simulink model "MCVT_Integration"  (R2020a) (continued)
== system MCVT_Integration/Sys5 - ECLSS/Active Thermal Control System (ATCS) == (continued)
  PID Motor speed Control : simulink/Continuous/PID Controller  Controller=PID  Form=Parallel  TimeDomain=Discrete-time  UseExternalTs=off  SampleTime=dt_5  IntegratorMethod=Backward Euler  FilterMethod=Forward Euler  ControllerParametersSource=internal  P=200  I=20  D=0  UseFilter=off  N=100  TunerSelectOption=Transfer Function Based (PID Tuner App)  InitialConditionSource=internal  InitialConditionForIntegrator=1  InitialConditionForFilter=0  DifferentiatorICPrevScaledInput=0  ExternalReset=none  TrackingMode=off  Kt=1  LimitOutput=on  UpperSaturationLimit=120  LowerSaturationLimit=0  LinearizeAsGain=off  AntiWindupMode=none  Kb=1  POutMin=[]  POutMax=[]  IOutMin=[]  IOutMax=[]  DOutMin=[]  DOutMax=[]  NOutMin=[]  NOutMax=[]  SumOutMin=[]  SumOutMax=[]  SaturationOutMin=[]  SaturationOutMax=[]  PParamMin=[]  PParamMax=[]  IParamMin=[]  IParamMax=[]  DParamMin=[]  DParamMax=[]  NParamMin=[]  NParamMax=[]  KbParamMin=[]  KbParamMax=[]  KtParamMin=[]  KtParamMax=[]  KbOutMin=[]  KbOutMax=[]  KtOutMin=[]  KtOutMax=[]  IntegratorOutMin=[]  IntegratorOutMax=[]  FilterOutMin=[]  FilterOutMax=[]  SumI1OutMin=[]  SumI1OutMax=[]  SumI2OutMin=[]  SumI2OutMax=[]  SumI3OutMin=[]  SumI3OutMax=[]  SumI4OutMin=[]  SumI4OutMax=[]  SumDOutMin=[]  SumDOutMax=[]  DifferentiatorOutMin=[]  DifferentiatorOutMax=[]  FilterDiffOutCoefMin=[]  FilterDiffOutCoefMax=[]  ReciprocalOutMin=[]  ReciprocalOutMax=[]  SumDenOutMin=[]  SumDenOutMax=[]  SumNumOutMin=[]  SumNumOutMax=[]  DivideOutMin=[]  DivideOutMax=[]  UdiffTsProdOutMin=[]  UdiffTsProdOutMax=[]  NTsProdOutMin=[]  NTsProdOutMax=[]  UintegralTsProdOutMin=[]  UintegralTsProdOutMax=[]  UngainTsProdOutMin=[]  UngainTsProdOutMax=[]  IntegratorContinuousStateAttributes=''  IntegratorStateMustResolveToSignalObject=off  IntegratorRTWStateStorageClass=Auto  FilterContinuousStateAttributes=''  FilterStateMustResolveToSignalObject=off  FilterRTWStateStorageClass=Auto  ParallelPVariant=InternalParameters  IdealPVariant=Disabled  IVariant=InternalParameters  DVariant=InternalParameters  IntegratorVariant=Discrete  SatVariant=Enabled  AWVariant=Passthrough  PCopyVariant=Disabled  TRVariant=Disabled  FdbkBlocksVariant=Forward  IdealPFdbkVariant=Disabled  SatFdbkVariant=Disabled  DerivativeFilterVariant=Differentiator  NVariant=Passthrough  NCopyVariant=Disabled_SignalSpec  FilterICVariant=InternalICsDifferentiator  IntegratorICVariant=InternalICs  ExternalResetVariant=Disabled  TRSumVariant=Passthrough  SumFdbkVariant=Disabled  SumVariant=Sum_PID  TsampFilterVariant=InternalTs  TsampNgainVariant=Passthrough  TsampIntegralVariant=Passthrough
  Scope : Scope  Commented=on  NumInputPorts=2
  Scope1 : Scope  Commented=on
  Scope2 : Scope  Commented=on
  Scope3 : Scope  Commented=on
  Scope4 : Scope
  Sensors : MATLAB Function  TreatAsAtomicUnit=on
  Sensors1 : MATLAB Function  TreatAsAtomicUnit=on
  Sum : Sum  Inputs=+-
  Sum1 : Sum  Inputs=|+-
  Sum2 : Sum
  Sys5_int_air_properties : Constant  Value=input_air
  Sys5_int_compressor_parameters : Constant  Value=input_CP
  Sys5_int_condenser_parameters : Constant  Value=input_Cond
  Sys5_int_discretization_information : Constant  Value=input_discretization
  Sys5_int_evaporator_parameters : Constant  Value=input_Evap
  Sys5_int_expansion_vavle_parameters : Constant  Value=input_EV
  Sys5_int_radiator_loop_parameters : Constant  Value=input_radiatorLoop
  Variable set temperature : MATLAB Function  TreatAsAtomicUnit=on  SystemSampleTime=dt_5
  Vavle opening control : MATLAB Function  TreatAsAtomicUnit=on
  on and off dynamic enforcer : MATLAB Function  TreatAsAtomicUnit=on
  tempadjust : MATLAB Function  TreatAsAtomicUnit=on  SystemSampleTime=dt_5
  Sys5_out_sensor_data_Tfs [C] : Outport  VectorParamsAs1DForOutWhenUnconnected=off
  Sys5_out_sensor_data_Trs [C] : Outport  Port=2  VectorParamsAs1DForOutWhenUnconnected=off
  Sys5_out_power_request [kW] : Outport  Port=3
  Sys5_out_power consumption [kW] : Outport  Port=4  VectorParamsAs1DForOutWhenUnconnected=off
  Sys5_out_heating_energy [kW] : Outport  Port=5  VectorParamsAs1DForOutWhenUnconnected=off
  Sys5_out_airflow [m^3/s] : Outport  Port=6  VectorParamsAs1DForOutWhenUnconnected=off
  Sys5_out_evaporator temperature [C] : Outport  Port=7  VectorParamsAs1DForOutWhenUnconnected=off
  "Sys5_out_paint Health State (0: clean, 1: full damage)" : Outport  Port=8
  "Sys5_out_dust Health State (0: clean, 1: full damage)" : Outport  Port=9
  Sys5_Out_Damage_Information : Outport  Port=10
  wires:
  Sys5_int_evaporator_parameters/1 -> ATCS/4(input_Evap)
  Sys5_int_compressor_parameters/1 -> ATCS/6(input_CP)
  Sys5_int_condenser_parameters/1 -> ATCS/5(input_Cond)
  ATCS/12(Ye) -> Memory9/1
  Memory1/1 -> ATCS/1(Xk)
  Sys5_int_radiator_loop_parameters/1 -> ATCS/8(input_RL)
  Sys5_int_discretization_information/1 -> ATCS/3(input_discretization)
  Sys5_int_air_properties/1 -> ATCS/7(input_air)
  ATCS/1(X) -> Memory1/1
  Sum/1 -> PID Motor speed Control/1
  Sys5_in_solar radiation [W/m^2]/1 -> [A]
  ATCS/9(Tair_out) -> Sys5_out_evaporator temperature [C]/1
  Vavle opening control/1(input_EV) -> ATCS/14(input_EV)
  Liquid fraction setpoint/1 -> Vavle opening control/2(Ye_set)
  Memory9/1 -> Vavle opening control/1(Ye)
  Sys5_int_expansion_vavle_parameters/1 -> Vavle opening control/3("input_EV ")
  Variable set temperature/2(set) -> Memory4/1
  Memory4/1 -> Variable set temperature/5("set ")
  Faulty Panels/3(faulty panels) -> ATCS/9(input_dust)
  ATCS/3(Pc) -> on and off dynamic enforcer/2(Pc)
  ATCS/4(Tfs) -> Sensors/1(Tfs)
  Sensors/1(TTfs) -> Sys5_out_sensor_data_Tfs [C]/1
  ATCS/5(Trs) -> Sensors1/1(Trs)
  Sensors1/1(TTrs) -> Sys5_out_sensor_data_Trs [C]/1
  Heater_capacity (kW)/1 -> MATLAB Function6/3(Qh)
  ATCS/10(WCP) -> Add/1, Scope2/1, Sum2/1, Variable set temperature/1(Pd)
  MATLAB Function6/2(Ph) -> [D]
  Add/1 -> Sys5_out_power consumption [kW]/1
  PID Motor speed Control/1 -> ATCS/13(nCP), Scope1/1
  Sys5_in_airflow through the evaporator [m^3/s]/1 -> ATCS/12(Vair_in)
  Variable set temperature/1(Tset) -> Memory2/1, Memory3/1
  on and off dynamic enforcer/1(Vop) -> Memory6/1
  ATCS/2(Pe) -> on and off dynamic enforcer/1(Pe)
  Memory6/1 -> ATCS/2(Vop)
  Faulty Panels/1("Paint Health State(0: clean, 1:full damage)") -> [F]
  Faulty Panels/2("Dust Health State (0: clean, 1:full damage)") -> [G]
  "Sys5_in_dust repairing agent (1:active, 0:inactive)"/1 -> Faulty Panels/2("Dust repairing agent (1: active, 0: inactive)")
  "Sys5_in_paint repairing agent (1:active, 0:inactive)"/1 -> Faulty Panels/3("Paint repairing agent (1: active, 0: inactive)")
  Sum1/1 -> Heater PID Controller/1
  Heater PID Controller/1 -> MATLAB Function6/1(Ps)
  Sys5_in_limited power supply [kW]/1 -> MATLAB Function6/2(lim_supply), Variable set temperature/3(Ps), Scope4/1
  Memory3/1 -> Variable set temperature/4("Tset ")
  Sys5_in_impact (1-4)/1 -> Faulty Panels/4(Impact)
  MATLAB Function6/1(P_request) -> Sum2/2
  Sum2/1 -> Sys5_out_power_request [kW]/1
  Sys5_in_Tset [C]/1 -> tempadjust/2(Tset), Variable set temperature/2(Tset0)
  Fan switch/1 -> [E]
  Gain/1 -> Faulty Panels/1(Sys5_in_Dust deposition rate (g/cm^2))
  Sys5_in_indoor temperature [C]/1 -> tempadjust/1(x)  "Indoor Temp"
  Clock/1 -> tempadjust/3(t)
  Discrete-Time Integrator/1 -> Gain/1
  Sys5_in_dust_Input [g/cm^2/s]/1 -> Discrete-Time Integrator/1
  Fan speed on (m^3/s)/1 -> Fan switch/1
  Fan speed off (m^3/s)/1 -> Fan switch/3
  [A] -> ATCS/10(Fs)
  tempadjust/1(y) -> [B]  "Indoor Temp"
  [B] -> ATCS/11(Tair_in)
  [B] -> Scope/2, Sum/1
  [B] -> Sum1/2
  [B] -> Fan switch/2
  Memory2/1 -> [C]  "Tset"
  [C] -> Sum1/1
  [C] -> Scope/1, Sum/2
  [D] -> Sys5_out_heating_energy [kW]/1
  [D] -> Scope3/1, Add/2
  [E] -> Sys5_out_airflow [m^3/s]/1
  [F] -> "Sys5_out_paint Health State (0: clean, 1: full damage)"/1
  [G] -> "Sys5_out_dust Health State (0: clean, 1: full damage)"/1
  Sys5_Repair_Status/1 -> Bus Selector/1
  Bus Selector/1 -> [Repair_ECLSS_Dust]  "<Repair_ECLSS_Dust>"
  Bus Selector/2 -> [Repair_ECLSS_Paint]  "<Repair_ECLSS_Paint>"
  [Repair_ECLSS_Dust] -> Faulty Panels/5(Repair_ECLSS_Dust)
  [Repair_ECLSS_Paint] -> Faulty Panels/6(Repair_ECLSS_Paint)
  Faulty Panels/4(Sys5_Out_Damage_Information) -> [Sys5_Out_Damage_Information]
  [Sys5_Out_Damage_Information] -> Sys5_Out_Damage_Information/1

== system MCVT_Integration/Sys5 - ECLSS/Human//System 5 ==
  dt : Inport
  activity_id : Inport  Port=2
  Location_id : Inport  Port=3
  Human Heat Model V2 : MATLAB Function  TreatAsAtomicUnit=on
  heat_profile_final : Outport
  r : Outport  Port=2
  theta : Outport  Port=3
  wires:
  Human Heat Model V2/1(heat_profile_final) -> heat_profile_final/1
  Location_id/1 -> Human Heat Model V2/3(Location_id)
  Human Heat Model V2/2(r) -> r/1
  Human Heat Model V2/3(theta) -> theta/1
  dt/1 -> Human Heat Model V2/1(dt)
  activity_id/1 -> Human Heat Model V2/2(activity_id)

== system MCVT_Integration/Sys5 - ECLSS/Interior Pressure Control System (IPCS) ==
  note: Inputs
  note: Controller
  note: Outputs
  Sys5_in_setpoint_pressure [Pa] : Inport
  Sys 5_in_interior pressure [Pa] : Inport  Port=2
  Sys5_in_faulty_valve [-] : Inport  Port=3
  Air storage tank volume : Constant  Value=ECLSS_Vst
  Area of Flow Restrictor : Constant  Value=ECLSS_IPS_restrictor.cross_area
  Area of Relief valve : Constant  Value=Aref
  Gain1 : Gain  Gain=-1
  High pressure stored air : MATLAB Function  TreatAsAtomicUnit=on  SystemSampleTime=dt_5
  Memory5 : Memory  InitialCondition=Ps0  InheritSampleTime=on
  Memory6 : Memory  InheritSampleTime=on
  "PID Controller Storage Control Valve " : simulink/Continuous/PID Controller  Controller=PID  Form=Parallel  TimeDomain=Discrete-time  UseExternalTs=off  SampleTime=dt_5  IntegratorMethod=Forward Euler  FilterMethod=Forward Euler  ControllerParametersSource=internal  P=1e-10  I=1e-11  D=0  UseFilter=on  N=100  TunerSelectOption=Transfer Function Based (PID Tuner App)  InitialConditionSource=internal  InitialConditionForIntegrator=0  InitialConditionForFilter=0  DifferentiatorICPrevScaledInput=0  ExternalReset=none  TrackingMode=off  Kt=1  LimitOutput=off  UpperSaturationLimit=A_flow_restrictor  LowerSaturationLimit=0  LinearizeAsGain=on  AntiWindupMode=none  Kb=1  POutMin=[]  POutMax=[]  IOutMin=[]  IOutMax=[]  DOutMin=[]  DOutMax=[]  NOutMin=[]  NOutMax=[]  SumOutMin=[]  SumOutMax=[]  SaturationOutMin=[]  SaturationOutMax=[]  PParamMin=[]  PParamMax=[]  IParamMin=[]  IParamMax=[]  DParamMin=[]  DParamMax=[]  NParamMin=[]  NParamMax=[]  KbParamMin=[]  KbParamMax=[]  KtParamMin=[]  KtParamMax=[]  KbOutMin=[]  KbOutMax=[]  KtOutMin=[]  KtOutMax=[]  IntegratorOutMin=[]  IntegratorOutMax=[]  FilterOutMin=[]  FilterOutMax=[]  SumI1OutMin=[]  SumI1OutMax=[]  SumI2OutMin=[]  SumI2OutMax=[]  SumI3OutMin=[]  SumI3OutMax=[]  SumI4OutMin=[]  SumI4OutMax=[]  SumDOutMin=[]  SumDOutMax=[]  DifferentiatorOutMin=[]  DifferentiatorOutMax=[]  FilterDiffOutCoefMin=[]  FilterDiffOutCoefMax=[]  ReciprocalOutMin=[]  ReciprocalOutMax=[]  SumDenOutMin=[]  SumDenOutMax=[]  SumNumOutMin=[]  SumNumOutMax=[]  DivideOutMin=[]  DivideOutMax=[]  UdiffTsProdOutMin=[]  UdiffTsProdOutMax=[]  NTsProdOutMin=[]  NTsProdOutMax=[]  UintegralTsProdOutMin=[]  UintegralTsProdOutMax=[]  UngainTsProdOutMin=[]  UngainTsProdOutMax=[]  IntegratorContinuousStateAttributes=''  IntegratorStateMustResolveToSignalObject=off  IntegratorRTWStateStorageClass=Auto  FilterContinuousStateAttributes=''  FilterStateMustResolveToSignalObject=off  FilterRTWStateStorageClass=Auto  ParallelPVariant=InternalParameters  IdealPVariant=Disabled  IVariant=InternalParameters  DVariant=InternalParameters  IntegratorVariant=Discrete  SatVariant=Disabled  AWVariant=Passthrough  PCopyVariant=Disabled  TRVariant=Disabled  FdbkBlocksVariant=Forward  IdealPFdbkVariant=Disabled  SatFdbkVariant=Disabled  DerivativeFilterVariant=ForwardEulerFilter  NVariant=InternalParameters  NCopyVariant=Disabled  FilterICVariant=InternalICsFilter  IntegratorICVariant=InternalICs  ExternalResetVariant=Disabled  TRSumVariant=Passthrough  SumFdbkVariant=Disabled  SumVariant=Sum_PID  TsampFilterVariant=InternalTs  TsampNgainVariant=Passthrough  TsampIntegralVariant=Passthrough
  Power consumption Calculator : MATLAB Function  TreatAsAtomicUnit=on
  Relief Valve Control : MATLAB Function  TreatAsAtomicUnit=on
  Restrictor : MATLAB Function  TreatAsAtomicUnit=on
  Saturation : Saturate  UpperLimit=ECLSS_IPS_restrictor.cross_area  LowerLimit=0
  Saturation1 : Saturate  UpperLimit=Aref  LowerLimit=0
  Scope : Scope
  Scope1 : Scope  NumInputPorts=2
  Scope2 : Scope  NumInputPorts=2
  Scope3 : Scope  NumInputPorts=2
  Sum : Sum  Inputs=|+-
  Sys5.2_int_valve parameter : Constant  Value=Kref
  Timestep for system 5 : Constant  Value=dt_5
  Sys5_out_air_released [kg/s] : Outport  VectorParamsAs1DForOutWhenUnconnected=off
  Sys5_out_air_supply [kg/s] : Outport  Port=2  VectorParamsAs1DForOutWhenUnconnected=off
  Sys5_out_consumed_power [kW] : Outport  Port=3
  wires:
  Power consumption Calculator/1(power) -> Sys5_out_consumed_power [kW]/1
  Saturation/1 -> Power consumption Calculator/1(Fr_now), Scope2/1, Restrictor/2(Afr)  "effective Cross-sectional Area"
  Area of Relief valve/1 -> Scope3/2, Power consumption Calculator/4(Rel_100)
  "PID Controller Storage Control Valve "/1 -> Saturation/1, Scope/1, Gain1/1
  Sys5.2_int_valve parameter/1 -> Relief Valve Control/2(Kref)
  Sum/1 -> "PID Controller Storage Control Valve "/1
  Saturation1/1 -> Scope3/1, Power consumption Calculator/3(Rel_now), Relief Valve Control/1(Aref)  "effective Cross-sectional Area"
  High pressure stored air/1(Ps) -> Memory5/1, Restrictor/1(Ps)
  Memory6/1 -> High pressure stored air/3(ms)
  Area of Flow Restrictor/1 -> Scope2/2, Power consumption Calculator/2(Fr_100)
  Memory5/1 -> High pressure stored air/1(Psk)
  Timestep for system 5/1 -> High pressure stored air/2(dt)
  Relief Valve Control/1(m_rel) -> Sys5_out_air_released [kg/s]/1
  Gain1/1 -> Saturation1/1
  Sys5_in_faulty_valve [-]/1 -> [B]
  Sys5_in_setpoint_pressure [Pa]/1 -> Sum/1, Scope1/1
  Air storage tank volume/1 -> High pressure stored air/4(ECLSS_Vst)
  Restrictor/1(ms) -> Sys5_out_air_supply [kg/s]/1, Memory6/1
  Sys 5_in_interior pressure [Pa]/1 -> [A]
  [A] -> Scope1/2, Sum/2
  [A] -> Relief Valve Control/3(Pint)
  [A] -> Restrictor/3(Pint)
  [B] -> Restrictor/4(Vop)

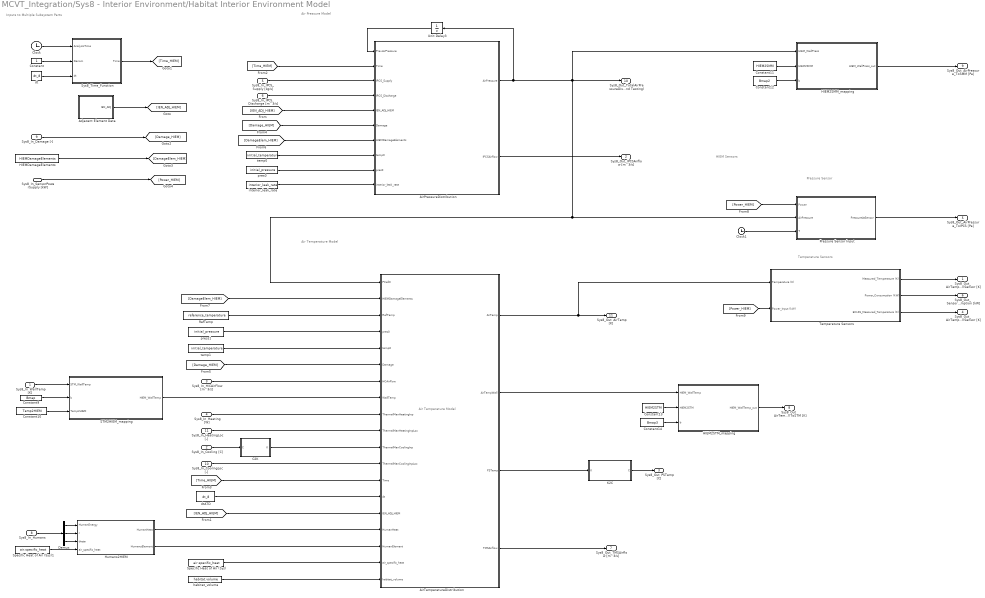
== system MCVT_Integration/Sys8 - Interior Environment/Habitat Interior Environment Model ==
  note: HIEM Sensors
  note: Inputs to Multiple Subsystem Parts
  note: Air Temperature Model
  note: Air Pressure Model
  note: Air Temperature Model
  note: Temperature Sensors
  note: Pressure Sensor
  Sys8_In_WallTemp [K] : Inport
  Sys8_In_Cooling [C] : Inport  Port=2
  Sys8_In_H/CAirFlow [m^3/s] : Inport  Port=3
  Sys8_In_Heating [W] : Inport  Port=4
  Sys8_In_IPCS_Supply [kg/s] : Inport  Port=5
  Sys8_In_IPCS_Discharge [m^3/s] : Inport  Port=6
  Sys8_In_SensorPowerSupply [kW] : Inport  Port=7
  Sys8_In_Humans : Inport  Port=8
  Sys8_In_Damage [-] : Inport  Port=9
  Sys8_In_CoolingLoc [-] : Inport  Port=10
  Sys8_In_HeatingLoc [-] : Inport  Port=11
  Adjacent Element Data : MATLAB Function  TreatAsAtomicUnit=on  SystemSampleTime=dt_8
  AirPressureDistribution : MATLAB Function  TreatAsAtomicUnit=on
  AirTemperatureDistribution : MATLAB Function  TreatAsAtomicUnit=on
  C2K : MATLAB Function  TreatAsAtomicUnit=on
  Clock : Clock
      settings: Decimation=5
  Clock1 : Clock
  Constant : Constant  SampleTime=dt_8
  Constant10 : Constant  Value=Temp2HIEM
  Constant11 : Constant  Value=HIEM2SMM
  Constant12 : Constant  Value=Bmap2
  Constant13 : Constant  Value=HIEM2STM
  Constant14 : Constant  Value=Bmap3
  Constant9 : Constant  Value=Bmap
  Demux : Demux  Outputs=3
  HIEM2SMM_mapping : MATLAB Function  TreatAsAtomicUnit=on
  HIEM2STM_mapping : MATLAB Function  TreatAsAtomicUnit=on
  HIEMDamageElements : Constant  Value=HIEMDamageElements
  Humans2HIEM : MATLAB Function  TreatAsAtomicUnit=on
  K2C : MATLAB Function  TreatAsAtomicUnit=on
  Pressure Sensor Input : MATLAB Function  TreatAsAtomicUnit=on  SystemSampleTime=dt_8
  RefTemp : Constant  Value=reference_temperature
  STM2HIEM_mapping : MATLAB Function  TreatAsAtomicUnit=on
  Specific Heat of Air (Sys) : Constant  Value=air.specific_heat
  Specific Heat of Air (Sys)1 : Constant  Value=air.specific_heat
  Sys8_Time_Function : MATLAB Function  TreatAsAtomicUnit=on  SystemSampleTime=dt_8
  Temperature Sensors : Subsystem
  Unit Delay3 : UnitDelay  InitialCondition=ones(112,1)*100000  SampleTime=dt_8  HasFrameUpgradeWarning=on
  dt : Constant  Value=dt_8
  dtATD : Constant  Value=dt_8
  habitat_volume : Constant  Value=habitat.volume
  interior_leak_rate : Constant  Value=interior_leak_rate
  pres0 : Constant  Value=initial_pressure
  press1 : Constant  Value=initial_pressure
  temp0 : Constant  Value=initial_temperature
  temp1 : Constant  Value=initial_temperature
  Sys8_Out_AirTempWallSensor [K] : Outport  PortDimensions=[3 1]  VectorParamsAs1DForOutWhenUnconnected=off
  Sys8_Out_IPCSAirflow [m^3/s] : Outport  Port=2  VectorParamsAs1DForOutWhenUnconnected=off
  Sys8_Out_PSTemp [C] : Outport  Port=3  OutDataTypeStr=double  PortDimensions=1  VectorParamsAs1DForOutWhenUnconnected=off
  Sys8_Out_AirTempECLSSWallSensor [K] : Outport  Port=4  VectorParamsAs1DForOutWhenUnconnected=off
  Sys8_Out_AirPressure_ToIPCS [Pa] : Outport  Port=5  VectorParamsAs1DForOutWhenUnconnected=off
  Sys8_Out_SensorPowerConsumption [kW] : Outport  Port=6  VectorParamsAs1DForOutWhenUnconnected=off
  Sys8_Out_TMSAirflow [m^3/s] : Outport  Port=7  VectorParamsAs1DForOutWhenUnconnected=off
  Sys8_Out_AirTempWallToSTM [K] : Outport  Port=8  VectorParamsAs1DForOutWhenUnconnected=off
  Sys8_Out_AirPressure_ToSMM [Pa] : Outport  Port=9  VectorParamsAs1DForOutWhenUnconnected=off
  Sys8_Out_TotalAirPressureDistribution (Plotting and Testing) : Outport  Port=10
  Sys8_Out_AirTemp [K] : Outport  Port=11  VectorParamsAs1DForOutWhenUnconnected=off
  wires:
  Clock/1 -> Sys8_Time_Function/1(AnalysisTime)
  Constant/1 -> Sys8_Time_Function/2(Denom)
  dt/1 -> Sys8_Time_Function/3(dt)
  Sys8_In_Cooling [C]/1 -> C2K/1(C)
  Sys8_In_WallTemp [K]/1 -> STM2HIEM_mapping/1(STM_WallTemp)
  Unit Delay3/1 -> AirPressureDistribution/1(PrevAirPressure)
  Sys8_In_CoolingLoc [-]/1 -> AirTemperatureDistribution/12(ThermalManCoolingInpLoc)
  Sys8_In_IPCS_Supply [kg/s]/1 -> AirPressureDistribution/3(IPCS_Supply)
  Sys8_In_IPCS_Discharge [m^3/s]/1 -> AirPressureDistribution/4(IPCS_Discharge)
  Pressure Sensor Input/1(PressureAtSensor) -> Sys8_Out_AirPressure_ToIPCS [Pa]/1
  C2K/1(K) -> AirTemperatureDistribution/11(ThermalManCoolingInp)
  K2C/1(C) -> Sys8_Out_PSTemp [C]/1
  dtATD/1 -> AirTemperatureDistribution/14(dt)
  AirTemperatureDistribution/1(AirTemp) -> Sys8_Out_AirTemp [K]/1, Temperature Sensors/1(Temperature (K))
  AirTemperatureDistribution/3(PSTemp) -> K2C/1(K)
  AirTemperatureDistribution/2(AirTempWall) -> HIEM2STM_mapping/1(HIEM_WallTemp)
  AirPressureDistribution/2(IPCSAirflow) -> Sys8_Out_IPCSAirflow [m^3/s]/1
  AirPressureDistribution/1(AirPressure) -> Unit Delay3/1, HIEM2SMM_mapping/1(HIEM_WallPress), Sys8_Out_TotalAirPressureDistribution (Plotting and Testing)/1, AirTemperatureDistribution/1(PCell0), Pressure Sensor Input/2(AirPressure)
  Sys8_In_H/CAirFlow [m^3/s]/1 -> AirTemperatureDistribution/7(HCAirFlow)
  Adjacent Element Data/1(IEN_ADJ) -> [IEN_ADJ_HIEM]
  Sys8_In_SensorPowerSupply [kW]/1 -> [Power_HIEM]
  Temperature Sensors/1(Measured_Temperature (K)) -> Sys8_Out_AirTempWallSensor [K]/1
  Temperature Sensors/2(Power_Consumption (kW)) -> Sys8_Out_SensorPowerConsumption [kW]/1
  AirTemperatureDistribution/4(TMSAirflow) -> Sys8_Out_TMSAirflow [m^3/s]/1
  HIEM2SMM_mapping/1(HIEM_WallPress_out) -> Sys8_Out_AirPressure_ToSMM [Pa]/1
  STM2HIEM_mapping/1(HIEM_WallTemp) -> AirTemperatureDistribution/8(WallTemp)
  HIEM2STM_mapping/1(HIEM_WallTemp_out) -> Sys8_Out_AirTempWallToSTM [K]/1
  Sys8_In_Humans/1 -> Demux/1
  Demux/2 -> Humans2HIEM/2(r)
  Demux/3 -> Humans2HIEM/3(theta)
  Demux/1 -> Humans2HIEM/1(HumanEnergy)
  Humans2HIEM/1(HumanHeat) -> AirTemperatureDistribution/16(HumanHeat)
  Humans2HIEM/2(HumansElement) -> AirTemperatureDistribution/17(HumanElement)
  Sys8_In_Heating [W]/1 -> AirTemperatureDistribution/9(ThermalManHeatingInp)
  Sys8_In_HeatingLoc [-]/1 -> AirTemperatureDistribution/10(ThermalManHeatingInpLoc)
  Sys8_Time_Function/1(Time) -> [Time_HIEM]
  Sys8_In_Damage [-]/1 -> [Damage_HIEM]
  Temperature Sensors/3(ECLSS_Measured_Temperature (K)) -> Sys8_Out_AirTempECLSSWallSensor [K]/1
  temp0/1 -> AirPressureDistribution/8(temp0)
  pres0/1 -> AirPressureDistribution/9(pres0)
  temp1/1 -> AirTemperatureDistribution/5(temp0)
  RefTemp/1 -> AirTemperatureDistribution/3(RefTemp)
  Constant9/1 -> STM2HIEM_mapping/2(b)
  Constant10/1 -> STM2HIEM_mapping/3(Temp2HIEM)
  Constant11/1 -> HIEM2SMM_mapping/2(HIEM2SMM)
  Constant12/1 -> HIEM2SMM_mapping/3(b)
  Constant14/1 -> HIEM2STM_mapping/3(b)
  Constant13/1 -> HIEM2STM_mapping/2(HIEM2STM)
  Clock1/1 -> Pressure Sensor Input/3(T)
  interior_leak_rate/1 -> AirPressureDistribution/10(interior_leak_rate)
  HIEMDamageElements/1 -> [DamageElem_HIEM]
  habitat_volume/1 -> AirTemperatureDistribution/19(habitat_volume)
  press1/1 -> AirTemperatureDistribution/4(pres0)
  Specific Heat of Air (Sys)/1 -> AirTemperatureDistribution/18(air_specific_heat)
  Specific Heat of Air (Sys)1/1 -> Humans2HIEM/4(air_specific_heat)
  [IEN_ADJ_HIEM] -> AirPressureDistribution/5(IEN_ADJ_HIEM)
  [IEN_ADJ_HIEM] -> AirTemperatureDistribution/15(IEN_ADJ_HIEM)
  [Time_HIEM] -> AirPressureDistribution/2(Time)
  [Time_HIEM] -> AirTemperatureDistribution/13(Time)
  [Damage_HIEM] -> AirPressureDistribution/6(Damage)
  [Damage_HIEM] -> AirTemperatureDistribution/6(Damage)
  [DamageElem_HIEM] -> AirPressureDistribution/7(HIEMDamageElements)
  [DamageElem_HIEM] -> AirTemperatureDistribution/2(HIEMDamageElements)
  [Power_HIEM] -> Temperature Sensors/2(Power_Input (kW))
  [Power_HIEM] -> Pressure Sensor Input/1(Power)

== system MCVT_Integration/Sys9 - Exterior Environment/Exterior Environment ==
  note: Moonquake
  note: Solar Irradiation
  note: Dust Accumulation
  note: Meteoroid Impact
  Sys9_in_Time : Inport
  Sys9_in_InclinationAngle : Inport  Port=2
  Sys9_in_Magnitude : Inport  Port=3
  3D Solar Irradiation model (v9) 1 : MATLAB Function  TreatAsAtomicUnit=on
  Constant6 : Constant  Value=0
  Delay : Delay  DelayLength=Sys9_int_EqStart/dt_9  InitialCondition=0
  Delay1 : Delay  DelayLength=Sys9_int_EqStart/dt_9  InitialCondition=0
  Demux : Demux  Outputs=3
  Determine_Damage_Case : MATLAB Function  TreatAsAtomicUnit=on
  Dust : Subsystem
  ExhaustEvent : Subsystem
  Impact_Location : MATLAB Function  TreatAsAtomicUnit=on
  Landing Pad Parameters1 : Constant  Value=Sys9_int_L  SampleTime=dt_9
  MATLAB Function : MATLAB Function  TreatAsAtomicUnit=on
  Mapping function from Solar Irradiation to SPLT and STM : MATLAB Function  TreatAsAtomicUnit=on
  MeteoroidEvent : Subsystem
  Reads cartesian coordinates : MATLAB Function  TreatAsAtomicUnit=on
  SR_STM_ei_th : ToWorkspace  VariableName=SR_STM_ei_th  SampleTime=-1
  SR_STM_ei_th2 : ToWorkspace  VariableName=SR_STM_ei_th2  SampleTime=-1
  Start time : Constant  Value=Start_angle*6720
  Sys9_int_LandingPadDistance1 : Constant  Value=Sys9_int_D  SampleTime=dt_9
  Sys9_int_meteoroidEvent : Constant  Value=meteoroidDustDep  SampleTime=dt_9
  Sys9_int_nominalDustDep : Constant  Value=nominalDustDep  SampleTime=dt_9
  Unit Delay : UnitDelay  SampleTime=-1  HasFrameUpgradeWarning=on
  Unit Delay1 : UnitDelay  SampleTime=-1  HasFrameUpgradeWarning=on
  damage case : Constant  Value=damage_case_id  SampleTime=dt_9
  meteoroite time : Constant  Value=meteorite_impact_time  SampleTime=dt_9
  Sys9_out_MetCordX : Outport
  Sys9_out_MetCordY : Outport  Port=2
  Sys9_out_MetCordZ : Outport  Port=3
  Sys9_out_FI_index : Outport  Port=4
  Sys9_out_dmg_case : Outport  Port=5
  Sys9_out_DustAccumulationLunch : Outport  Port=6
  Sys9_out_SolarFlux : Outport  Port=7
  Sys9_out_SolarFluxSpatial : Outport  Port=8
  Sys9_out_GroundAccX : Outport  Port=9
  Sys9_out_GroundAccY : Outport  Port=10
  Sys9_out_GroundAccZ : Outport  Port=11
  wires:
  MATLAB Function/2(Ag_y) -> Delay1/1
  MATLAB Function/1(Ag_x) -> Delay/1
  Sys9_in_Magnitude/1 -> MATLAB Function/2(Magnitude)
  Delay1/1 -> Sys9_out_GroundAccY/1
  Delay/1 -> Sys9_out_GroundAccX/1
  Sys9_in_Time/1 -> Determine_Damage_Case/1(t), MATLAB Function/1(t), 3D Solar Irradiation model (v9) 1/1(intime)
  Constant6/1 -> Sys9_out_GroundAccZ/1
  Impact_Location/1(FI_Loc) -> Demux/1
  Demux/1 -> Sys9_out_MetCordX/1
  Demux/2 -> Sys9_out_MetCordY/1
  Demux/3 -> Sys9_out_MetCordZ/1
  3D Solar Irradiation model (v9) 1/1(xyz_SR) -> Mapping function from Solar Irradiation to SPLT and STM/2(xyz_SR)
  Reads cartesian coordinates/1(pos_out) -> Mapping function from Solar Irradiation to SPLT and STM/1(pos_out)
  3D Solar Irradiation model (v9) 1/3(SR_ECLSS) -> Sys9_out_SolarFlux/1
  MeteoroidEvent/1(meteoroidEvent) -> Dust/2(meteoroidEvent)
  ExhaustEvent/1(Out1) -> Dust/1(exhaustEvent)
  Landing Pad Parameters1/1 -> Dust/6(input_L)
  Sys9_int_LandingPadDistance1/1 -> Dust/5(d)
  Sys9_int_meteoroidEvent/1 -> Dust/4(meteoroidDustDep)
  Sys9_int_nominalDustDep/1 -> Dust/3(nominalDustDep)
  Dust/1(tao_total) -> Sys9_out_DustAccumulationLunch/1
  Unit Delay1/1 -> Determine_Damage_Case/5("FI_tend ")
  Unit Delay/1 -> Determine_Damage_Case/4(countp)
  Determine_Damage_Case/2(dmg_caseo) -> Sys9_out_dmg_case/1
  Determine_Damage_Case/1(FI_index) -> Sys9_out_FI_index/1
  meteoroite time/1 -> Determine_Damage_Case/3(mtime)
  damage case/1 -> Determine_Damage_Case/2(dmg_case)
  Determine_Damage_Case/3(count) -> Unit Delay/1
  Determine_Damage_Case/4(FI_tend) -> Unit Delay1/1
  Start time/1 -> 3D Solar Irradiation model (v9) 1/2(Sttime)
  Mapping function from Solar Irradiation to SPLT and STM/2(SR_STM_ei_th) -> SR_STM_ei_th/1
  3D Solar Irradiation model (v9) 1/4(SR_STM_ei_th2) -> SR_STM_ei_th2/1
  3D Solar Irradiation model (v9) 1/6(Sb_fin_vec) -> Mapping function from Solar Irradiation to SPLT and STM/3(Sb_tot)
  Mapping function from Solar Irradiation to SPLT and STM/1(SR_STM) -> Sys9_out_SolarFluxSpatial/1
